simulink model "PlanarModelandControl"  (R2026a)
settings: Solver=FixedStepDiscrete  StopTime=StopTime  FixedStep=Ts  MaxStep=0  RelTol=1e-5  AbsTol=1e-3
PostLoadFcn:
  | Controles_Ativos = 1;
  | Vx_Ref = 0;
  | Vx_init = 0;
  | t_default = [0; 10000];
  | Brake_Cmd = [t_default, [0; 0]];
  | Accel_Cmd = [t_default, [0; 0]];
  | Steer_Cmd = [t_default, [0; 0]];
  | Flag_CruiseControl = 0;
  | Ts = 0.001;
  | load('TC_LUT.mat')
InitFcn:
  | % Carrega parâmetros gerais (Massa, Ganhos PID, Geometria)
  | run('Parameters.m');
  | 
  | % Carrega as Tabelas (Lookup Tables)
  | run('curva_steering.m');
  | run('curva_tq_motor.m');
  | load('TC_LUT.mat')
model workspace (MAT data in the model file):
  A = 1.22
  Ack = 1
  Cd = 1.1
  Cl = -2
  Cs = [19075.58 25992.8 31792.64 35898.02 39044.37]
  Fz0x = -811.9083
  Fz0y = -210.9467
  Fz_cs = [222.41 444.82 667.23 889.64 1112.05]
  IAY = 0
  IAx = 0
  IAy = 0
  Im = 0.00033
  It = 0.0094
  Iw = 0.1578
  Iw_ = 0.7
  Iz_car = 180
  K_50_understeer = 0.005184
  K_Stability = 1
  K_oversteer = -0.001
  Kappa_F = 0
  Kappa_R = 0
  Km_FM = 110000
  Km_Fm = 110000
  Km_RM = 120000
  Km_Rm = 120000
  KphiARB_F = 0
  KphiARB_R = 11000
  KphiF = 17054.5
  KphiR = 40034.8
  Kphim_F = 137500
  Kphim_R = 48600
  L = 1.53
  M = 300
  Mgt = 11.72
  P = 68.9476
  P0 = 55.4852
  R = 0.2032
  Rcurva = 20
  Re = 0.2
  SR = 3
  TV_On = 1
  Ts = 0.001
  W = 2940
  Wf = 308.034
  Wr = 339.426
  Ws = 2099.34
  YLL = 1.11022302463e-16
  cx1 = 1.3668
  cy1 = 1.839
  dpi = 0.242630467224
  dx1 = 2.1568
  dx2 = -0.065814
  dx3 = 0.0011254
  dy1 = 2.453
  dy2 = -0.11197
  dy3 = 10.2899
  ex1 = -0.19815
  ex2 = -0.62529
  ex3 = 1.3396
  ex4 = 0.0029464
  ey1 = 0.96085
  ey2 = -0.28662
  ey3 = 0.14389
  ey4 = 4.9274
  ey5 = -70.7165
  g = 9.81
  hcg = 0.252
  hcgs = 0.202
  hrc_f = 0.056
  hrc_r = 0.084
  hs_f = 0.222
  hs_r = 0.222
  hx1 = -0.003859
  hx2 = -0.000374
  hy1 = -0.000403
  hy2 = -0.000261
  kx1 = -36.9039
  kx2 = 22.3857
  kx3 = 0.61844
  ky1 = -83.2526
  ky2 = -5.565
  ky3 = 0.88491
  ky4 = 2.9994
  ky5 = -99.9393
  ky6 = -3.4705
  ky7 = -0.91452
  lf = 0.8415
  lr = 0.6885
  mass_FL = 67.5
  mass_FR = 67.5
  mass_RL = 82.5
  mass_RR = 82.5
  mi = 2
  ms = 240
  pty1 = 0.005744
  pty2 = 19.1334
  px1 = -0.039964
  px2 = -0.15015
  px3 = -0.32528
  px4 = -0.063273
  py1 = 0.38578
  py2 = 1.2048
  py3 = -0.14544
  py4 = 0.092886
  py5 = -0.82901
  r = 9
  rhoar = 1.22
  s_f = 1
  s_r = 0.9
  tf = 1.25
  tr = 1.25
  vx1 = -0.11922
  vx2 = 0.002205
  vy1 = 0.032907
  vy2 = 0.018068
  vy3 = -1.0429
  vy4 = 0.53712
  x_fl = 0.8415
  x_fr = 0.8415
  x_rl = 0.6885
  x_rr = 0.6885
  xs_f = 0.826
  xs_r = 0.704
  y_fl = 0.6
  y_fr = 0.6
  y_rl = 0.59
  y_rr = 0.59
values from neighbouring files (symbols this model uses):
  GG_mode = 0    [autocross.m]    (also in teste.mat)
  Gr = 11.72    [Parameters.m]    (also in teste.mat)
  K_variavel = [-0.001, 0, 0.0008, 0.0012, 0.0015]    [Parameters.m]    (also in teste.mat = [-0.001 0 0.0008 0.0012 0.0015])
  Ki_data = [283117.9493, 114831.615, 51596.0519, 30556.5344, 17525.221, 11773.8301, 10567.5001]*0.008    [Parameters.m]    (also in teste.mat = [2264.9435944 918.65292 412.7684152 244.4522752 140.201768 9)
  Kp_data = [2877.5702, 2303.9999, 1972.0835, 1556.8775, 1258.2451, 1022.7085, 982.7861, ]*5    [Parameters.m]    (also in teste.mat = [14387.851 11519.9995 9860.4175 7784.3875 6291.2255 5113.542)
  Mz_maxF = [0 66.3 132.6 198.9 265.2 330.23 396.52 462.83 529.112 595.42 661.73 728.03 794.33 860.62 926.92 993.23 1058.25 1124.55 1190.85 1257.15 1323.45 1389.75 1456.05 1522.35 1588.65 1654.95]    [Parameters.m]    (also in teste.mat = [0 66.3 132.6 198.9 265.2 330.23 396.52 462.83 529.112 595.4)
  Mz_minF = [0 -66.3 -132.6 -198.9 -265.2 -330.23 -396.52 -462.83 -529.112 -595.42 -661.73 -728.03 -794.33 -860.62 -926.92 -993.23 -1058.25 -1124.55 -1190.85 -1257.15 -1323.45 -1389.75 -1456.05 -1522.35 -1588.65 -1654.95]    [Parameters.m]    (also in teste.mat = [0 -66.3 -132.6 -198.9 -265.2 -330.23 -396.52 -462.83 -529.1)
  pedal = [0 4 8 12 16 20 24 28 32 36 40 44 48 52 56 60 64 68 72 76 80 84 88 92 96 100]    [Parameters.m]    (also in teste.mat = [0 4 8 12 16 20 24 28 32 36 40 44 ... (26 elements, 1x26)])
  rpm_355_novo = [0 10050 10100 10140 10170 10210 10250 10280 10320 10360         10390 10420 10460 10620 10770 10920 11050 11170 11280         11380 11470 11550 11625 11680 11730 11775 11790 11800]    [curva_tq_motor.m]    (also in teste.mat = [0 10050 10100 10140 10170 10210 10250 10280 10320 10360 103)
  Steering_inner = [-29.018 ... (first row only)    [curva_steering.m]    (also in teste.mat = [-29.018 -25.595 -22.357 -19.265 -16.292 -13.415 -10.619 -7.)
  Steering_outer = [-25.111 ... (first row only)    [curva_steering.m]    (also in teste.mat = [-25.111 -22.589 -20.082 -17.585 -15.094 -12.604 -10.11 -7.6)
  steering_vol = [-105 ... (first row only)    [curva_steering.m]    (also in teste.mat = [-105 -94.5 -84 -73.5 -63 -52.5 -42 -31.5 -21 -10.5 0 10.5 .)
  torque_355_novo = [16.2 16.2 16.0 15.8 15.6 15.4 15.2 15.0 14.8 14.6            14.4 14.2 14.0 13.0 12.0 11.0 10.0 9.0 8.0            7.0 6.0 5.0 4.0 3.0 2.0 1.0 0.5 0.0]    [curva_tq_motor.m]    (also in teste.mat = [16.2 16.2 16 15.8 15.6 15.4 15.2 15 14.8 14.6 14.4 14.2 ...)
  Vx = [0, 12.5, 23.6, 30, 35]    [Parameters.m]    (also in teste.mat = [0 12.5 23.6 30 35])
  Vx_axis = [5, 10, 15, 20, 25, 30, 35]    [Parameters.m]    (also in teste.mat = [5 10 15 20 25 30 35])
undefined symbols (not defined in the file or in its folder): DiscIntNeverNeededParam, magnitude
libraries: simulink x5, fuzblock x4, sl_discontinuities x3, simulink_extras x3, slpidlib x1
(part 2 of 4)

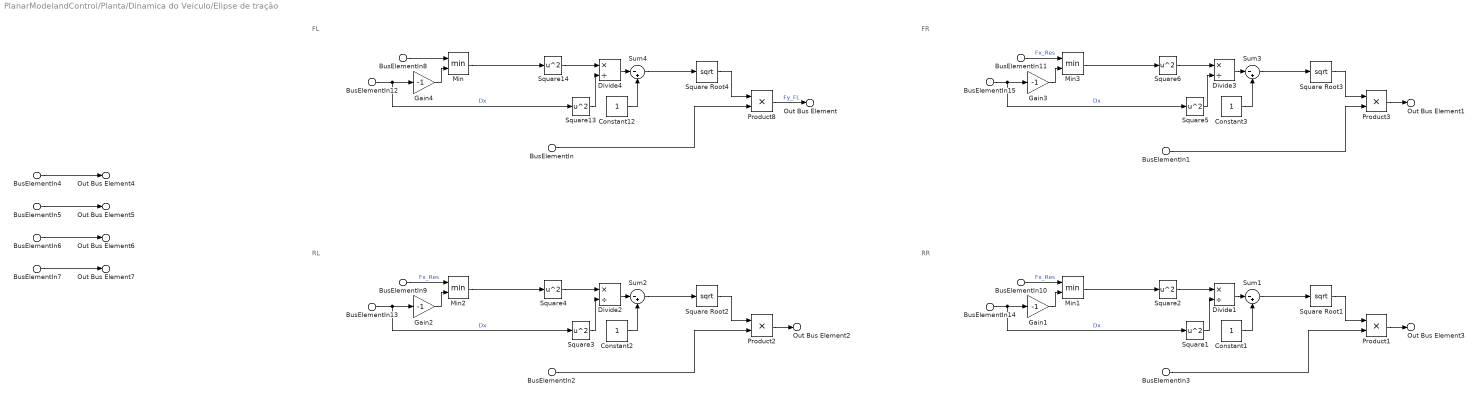
== system PlanarModelandControl/Planta/Dinamica do Veículo/Elipse de tração ==
  note: FL
  note: RR
  note: RL
  note: FR
  BusElementIn : Inport  PortName=Pacejka  Element=Fy0_FL
  BusElementIn1 : Inport  PortName=Pacejka  Element=Fy0_FR
  BusElementIn2 : Inport  PortName=Pacejka  Element=Fy0_RL
  BusElementIn3 : Inport  PortName=Pacejka  Element=Fy0_RR
  BusElementIn4 : Inport  PortName=Pacejka  Element=Fx0_FL
  BusElementIn8 : Inport  PortName=Pacejka  Element=Fx0_FL
  BusElementIn9 : Inport  PortName=Pacejka  Element=Fx0_RL
  BusElementIn6 : Inport  PortName=Pacejka  Element=Fx0_RL
  BusElementIn5 : Inport  PortName=Pacejka  Element=Fx0_FR
  BusElementIn11 : Inport  PortName=Pacejka  Element=Fx0_FR
  BusElementIn7 : Inport  PortName=Pacejka  Element=Fx0_RR
  BusElementIn10 : Inport  PortName=Pacejka  Element=Fx0_RR
  BusElementIn12 : Inport  PortName=Pacejka  Element=Dx_FL
  BusElementIn13 : Inport  PortName=Pacejka  Element=Dx_RL
  BusElementIn14 : Inport  PortName=Pacejka  Element=Dx_RR
  BusElementIn15 : Inport  PortName=Pacejka  Element=Dx_FR
  Constant{1..3,12} (4 alike) : Constant
  Divide{1..4} (4 alike) : Product  Inputs=*/
  Gain{1..4} (4 alike) : Gain  Gain=-1
  Min{,1..3} (4 alike) : MinMax  Inputs=2
  Product{1..3,8} (4 alike) : Product
  Square Root{1..4} (4 alike) : Sqrt
  Square{1..6,13..14} (8 alike) : Math  Operator=square
  Sum{1..4} (4 alike) : Sum  Inputs=|-+
  Out Bus Element : Outport  PortName=LatAndLongForces  Element=Fy_FL
  Out Bus Element3 : Outport  PortName=LatAndLongForces  Element=Fy_RR
  Out Bus Element2 : Outport  PortName=LatAndLongForces  Element=Fy_RL
  Out Bus Element1 : Outport  PortName=LatAndLongForces  Element=Fy_FR
  Out Bus Element4 : Outport  PortName=LatAndLongForces  Element=Fx_FL
  Out Bus Element5 : Outport  PortName=LatAndLongForces  Element=Fx_FR
  Out Bus Element6 : Outport  PortName=LatAndLongForces  Element=Fx_RL
  Out Bus Element7 : Outport  PortName=LatAndLongForces  Element=Fx_RR
  wires:
  Square13/1 -> Divide4/2
  Divide4/1 -> Sum4/1
  BusElementIn/1 -> Product8/2
  Square14/1 -> Divide4/1
  Constant12/1 -> Sum4/2
  BusElementIn8/1 -> Min/1
  Sum4/1 -> Square Root4/1
  BusElementIn12/1 -> Square13/1, Gain4/1  "Dx"
  Min/1 -> Square14/1
  Gain4/1 -> Min/2
  Product8/1 -> Out Bus Element/1  "Fy_FL"
  Square Root4/1 -> Product8/1
  BusElementIn10/1 -> Min1/1  "Fx_Res"
  Sum1/1 -> Square Root1/1
  Product1/1 -> Out Bus Element3/1
  Square2/1 -> Divide1/1
  Min1/1 -> Square2/1
  Constant1/1 -> Sum1/2
  Square1/1 -> Divide1/2
  Square Root1/1 -> Product1/1
  BusElementIn14/1 -> Square1/1, Gain1/1  "Dx"
  Gain1/1 -> Min1/2
  BusElementIn3/1 -> Product1/2
  Divide1/1 -> Sum1/1
  BusElementIn9/1 -> Min2/1  "Fx_Res"
  Sum2/1 -> Square Root2/1
  Product2/1 -> Out Bus Element2/1
  Square4/1 -> Divide2/1
  Min2/1 -> Square4/1
  Constant2/1 -> Sum2/2
  Square3/1 -> Divide2/2
  Square Root2/1 -> Product2/1
  BusElementIn13/1 -> Square3/1, Gain2/1  "Dx"
  Gain2/1 -> Min2/2
  BusElementIn2/1 -> Product2/2
  Divide2/1 -> Sum2/1
  BusElementIn11/1 -> Min3/1  "Fx_Res"
  Sum3/1 -> Square Root3/1
  Product3/1 -> Out Bus Element1/1
  Square6/1 -> Divide3/1
  Min3/1 -> Square6/1
  Constant3/1 -> Sum3/2
  Square5/1 -> Divide3/2
  Square Root3/1 -> Product3/1
  BusElementIn15/1 -> Square5/1, Gain3/1  "Dx"
  Gain3/1 -> Min3/2
  BusElementIn1/1 -> Product3/2
  Divide3/1 -> Sum3/1
  BusElementIn4/1 -> Out Bus Element4/1
  BusElementIn5/1 -> Out Bus Element5/1
  BusElementIn7/1 -> Out Bus Element7/1
  BusElementIn6/1 -> Out Bus Element6/1

== system PlanarModelandControl/Planta/Dinamica do Veículo/Frenagem ==
  Bus Element In6 : Inport  PortName=Driver  Element=BrakeCmd
  BusElementIn : Inport  Port=2  PortName=Movimento  Element=Vx_cg
  Constant : Constant  Value=0
  Gain : Gain  Gain=9508
  Switch : Switch  Criteria=u2 > Threshold  SaturateOnIntegerOverflow=off  InputSameDT=off
  Switch1 : Switch  Criteria=u2 > Threshold  SaturateOnIntegerOverflow=off  InputSameDT=off
  torque_máx_f : Gain  Gain=0.632
  torque_máx_r : Gain  Gain=1-0.632
  Bus Element Out2 : Outport  PortName=Brake  Element=Fbrake_FL
  Bus Element Out1 : Outport  PortName=Brake  Element=Fbrake_RL
  Bus Element Out3 : Outport  PortName=Brake  Element=Fbrake_FR
  Bus Element Out4 : Outport  PortName=Brake  Element=Fbrake_RR
  wires:
  torque_máx_r/1 -> Switch1/1
  Bus Element In6/1 -> Gain/1
  Constant/1 -> Switch1/3, Switch/3
  Switch1/1 -> Bus Element Out4/1, Bus Element Out1/1
  torque_máx_f/1 -> Switch/1
  Switch/1 -> Bus Element Out2/1, Bus Element Out3/1
  BusElementIn/1 -> Switch1/2, Switch/2
  Gain/1 -> torque_máx_r/1, torque_máx_f/1

== system PlanarModelandControl/Planta/Dinamica do Veículo/Load Transfer ==
  note: Lift
  In Bus Element : Inport  PortName=Movimento
  In Bus Element1 : Inport  PortName=Movimento
  Bus Element In5 : Inport  PortName=Movimento  Element=Vx_cg
  Gain10 : Gain  Gain=-1/2*rhoar*Cl*A
  Lateral Weight Transfer : Subsystem
  Longitudinal Weight Transfer : Subsystem
  Square : Math  Operator=square
  Total Normal Forces : Subsystem
  Out Bus Element : Outport  PortName=NormalForces
  wires:
  Square/1 -> Gain10/1
  Bus Element In5/1 -> Square/1
  In Bus Element/1 -> Longitudinal Weight Transfer/1(Movimento)
  In Bus Element1/1 -> Lateral Weight Transfer/1(Movimento)
  Longitudinal Weight Transfer/1(DeltaW_long) -> Total Normal Forces/2(DeltaW_long)
  Lateral Weight Transfer/1(DeltaW_lat) -> Total Normal Forces/3(DeltaW_lat)
  Gain10/1 -> Total Normal Forces/1(FLift)
  Total Normal Forces/1(NormalForces) -> Out Bus Element/1

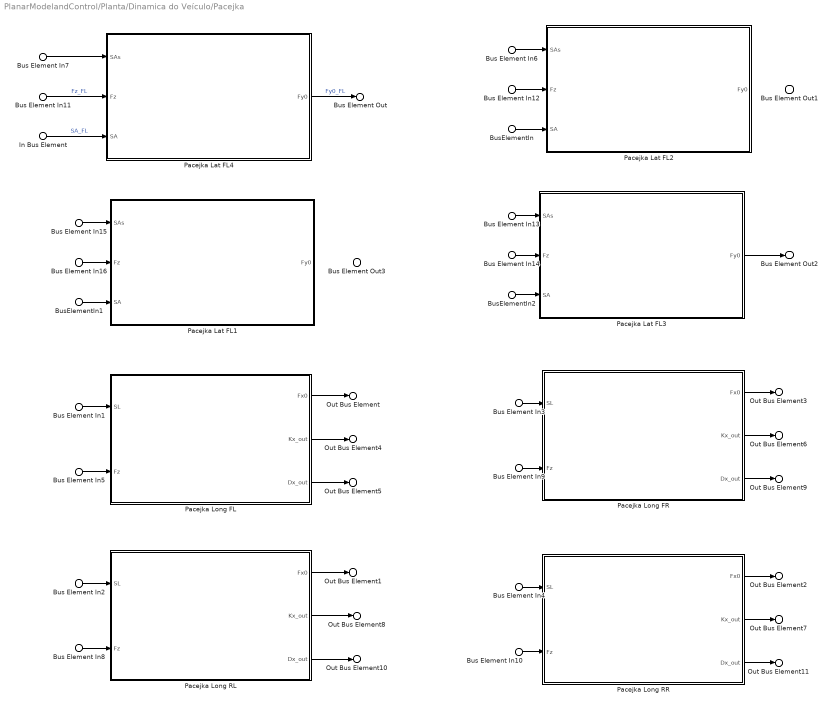
== system PlanarModelandControl/Planta/Dinamica do Veículo/Pacejka ==
  Bus Element In7 : Inport  PortName=RelaxionLength  Element=SAs_FL
  Bus Element In15 : Inport  PortName=RelaxionLength  Element=SAs_RL
  Bus Element In13 : Inport  PortName=RelaxionLength  Element=SAs_RR
  Bus Element In6 : Inport  PortName=RelaxionLength  Element=SAs_FR
  In Bus Element : Inport  Port=2  PortName=SlipAngles  Element=SA_FL
  BusElementIn : Inport  Port=2  PortName=SlipAngles  Element=SA_FR
  BusElementIn1 : Inport  Port=2  PortName=SlipAngles  Element=SA_RL
  BusElementIn2 : Inport  Port=2  PortName=SlipAngles  Element=SA_RR
  Bus Element In5 : Inport  Port=3  PortName=NormalForces  Element=Fz_FL
  Bus Element In11 : Inport  Port=3  PortName=NormalForces  Element=Fz_FL
  Bus Element In8 : Inport  Port=3  PortName=NormalForces  Element=Fz_RL
  Bus Element In16 : Inport  Port=3  PortName=NormalForces  Element=Fz_RL
  Bus Element In10 : Inport  Port=3  PortName=NormalForces  Element=Fz_RR
  Bus Element In14 : Inport  Port=3  PortName=NormalForces  Element=Fz_RR
  Bus Element In9 : Inport  Port=3  PortName=NormalForces  Element=Fz_FR
  Bus Element In12 : Inport  Port=3  PortName=NormalForces  Element=Fz_FR
  Bus Element In1 : Inport  Port=4  PortName=WheelSlips  Element=slip_long_FL
  Bus Element In3 : Inport  Port=4  PortName=WheelSlips  Element=slip_long_FR
  Bus Element In4 : Inport  Port=4  PortName=WheelSlips  Element=slip_long_RR
  Bus Element In2 : Inport  Port=4  PortName=WheelSlips  Element=slip_long_RL
  Pacejka Lat FL1 : Subsystem
  Pacejka Lat FL2 : Subsystem
  Pacejka Lat FL3 : Subsystem
  Pacejka Lat FL4 : Subsystem
  "Pacejka Long FL " : Subsystem
  Pacejka Long FR : Subsystem
  Pacejka Long RL : Subsystem
  Pacejka Long RR : Subsystem
  Bus Element Out : Outport  PortName=Pacejka  Element=Fy0_FL
  Out Bus Element : Outport  PortName=Pacejka  Element=Fx0_FL
  Out Bus Element1 : Outport  PortName=Pacejka  Element=Fx0_RL
  Out Bus Element2 : Outport  PortName=Pacejka  Element=Fx0_RR
  Out Bus Element3 : Outport  PortName=Pacejka  Element=Fx0_FR
  Out Bus Element4 : Outport  PortName=Pacejka  Element=Kx_FL
  Out Bus Element5 : Outport  PortName=Pacejka  Element=Dx_FL
  Out Bus Element8 : Outport  PortName=Pacejka  Element=Kx_RL
  Out Bus Element7 : Outport  PortName=Pacejka  Element=Kx_RR
  Out Bus Element6 : Outport  PortName=Pacejka  Element=Kx_FR
  Out Bus Element10 : Outport  PortName=Pacejka  Element=Dx_RL
  Out Bus Element11 : Outport  PortName=Pacejka  Element=Dx_RR
  Out Bus Element9 : Outport  PortName=Pacejka  Element=Dx_FR
  Bus Element Out2 : Outport  PortName=Pacejka  Element=Fy0_RR
  Bus Element Out1 : Outport  PortName=Pacejka  Element=Fy0_FR
  Bus Element Out3 : Outport  PortName=Pacejka  Element=Fy0_RL
  wires:
  Pacejka Lat FL4/1(Fy0) -> Bus Element Out/1  "Fy0_FL"
  "Pacejka Long FL "/1(Fx0) -> Out Bus Element/1
  Pacejka Long RL/1(Fx0) -> Out Bus Element1/1
  Pacejka Long RR/1(Fx0) -> Out Bus Element2/1
  Pacejka Long FR/1(Fx0) -> Out Bus Element3/1
  Bus Element In2/1 -> Pacejka Long RL/1(SL)
  Bus Element In3/1 -> Pacejka Long FR/1(SL)
  Bus Element In4/1 -> Pacejka Long RR/1(SL)
  Bus Element In1/1 -> "Pacejka Long FL "/1(SL)
  Bus Element In5/1 -> "Pacejka Long FL "/2(Fz)
  "Pacejka Long FL "/2(Kx_out) -> Out Bus Element4/1
  "Pacejka Long FL "/3(Dx_out) -> Out Bus Element5/1
  Bus Element In8/1 -> Pacejka Long RL/2(Fz)
  Bus Element In9/1 -> Pacejka Long FR/2(Fz)
  Bus Element In10/1 -> Pacejka Long RR/2(Fz)
  Pacejka Long FR/2(Kx_out) -> Out Bus Element6/1
  Pacejka Long RR/2(Kx_out) -> Out Bus Element7/1
  Pacejka Long RL/2(Kx_out) -> Out Bus Element8/1
  Pacejka Long FR/3(Dx_out) -> Out Bus Element9/1
  Pacejka Long RL/3(Dx_out) -> Out Bus Element10/1
  Pacejka Long RR/3(Dx_out) -> Out Bus Element11/1
  Bus Element In11/1 -> Pacejka Lat FL4/2(Fz)  "Fz_FL"
  Bus Element In7/1 -> Pacejka Lat FL4/1(SAs)
  Bus Element In12/1 -> Pacejka Lat FL2/2(Fz)
  ? -> Bus Element Out1/1
  Bus Element In6/1 -> Pacejka Lat FL2/1(SAs)
  Bus Element In14/1 -> Pacejka Lat FL3/2(Fz)
  Pacejka Lat FL3/1(Fy0) -> Bus Element Out2/1
  Bus Element In13/1 -> Pacejka Lat FL3/1(SAs)
  Bus Element In16/1 -> Pacejka Lat FL1/2(Fz)
  ? -> Bus Element Out3/1
  Bus Element In15/1 -> Pacejka Lat FL1/1(SAs)
  In Bus Element/1 -> Pacejka Lat FL4/3(SA)  "SA_FL"
  BusElementIn2/1 -> Pacejka Lat FL3/3(SA)
  BusElementIn/1 -> Pacejka Lat FL2/3(SA)
  BusElementIn1/1 -> Pacejka Lat FL1/3(SA)

== system PlanarModelandControl/Planta/Dinamica do Veículo/Relaxation Length ==
  note: Sigma - Relaxation Length
  Bus Element In1 : Inport  PortName=SlipAnges
  BusElementIn : Inport  Port=2  PortName=NormalForces  Element=Fz_FL
  BusElementIn1 : Inport  Port=2  PortName=NormalForces  Element=Fz_RL
  BusElementIn2 : Inport  Port=2  PortName=NormalForces  Element=Fz_RR
  BusElementIn3 : Inport  Port=2  PortName=NormalForces  Element=Fz_FR
  Bus Element In3 : Inport  Port=3  PortName=omega
  Atan{,1..3} (4 alike) : Trigonometry  Operator=atan
  Gain{,2,4,6} (4 alike) : Gain  Gain=1/(pty2*Fz0y)
  Gain{1,3,5,7} (4 alike) : Gain  Gain=2
  Gain{8..11} (4 alike) : Gain  Gain=pty1*Fz0y
  Sin{,1..3} (4 alike) : Trigonometry
  Subsystem : Subsystem
      settings: PortSchema="{\"entries\":[{\"content\":{\"sides\":[{\"content\":{\"connectorIds\":[\"In1\",\"In2\",\"In3\",\"In4\"]},\"type\":\"ConnectorPlacement.EquallySpacedRectSide\",\"uuid\":\"7f4a3b79-0cec-4aed-ad23-d33dc035a0be\"},{\"content\":{\"connectorIds\":[\"Out1\"],\"side\":\"RIGHT\"},\"type\":\"ConnectorPlacement.EquallySpacedRectSide\",\"uuid\":\"18b0376e-fcdd-4b64-8606-1eed368224ef\"},{\"content\":{\"connectorIds\":[\"In5\",\"In6\"],\"side\":\"TOP\"},\"type\":\"ConnectorPlacement.EquallySpacedRectSide\",\"uuid\":\"a3b43353-e9b4-4b33-921b-3423b398b207\"}]},\"type\":\"ConnectorPlacement.EquallySpacedConnector\",\"uuid\":\"fde8bdbb-5266-4a31-b332-536ff94eb04f\"}],\"packageUris\":[\"http://schema.mathworks.com/mf0/Connector/R2020b\"],\"version\":\"1.0\"}"
  Out Bus Element : Outport  PortName=RelaxionLength
  wires:
  Gain3/1 -> Sin1/1
  Gain4/1 -> Atan2/1
  Gain/1 -> Atan/1
  Gain5/1 -> Sin2/1
  Sin2/1 -> Gain10/1
  BusElementIn2/1 -> Gain6/1
  Gain2/1 -> Atan1/1
  Atan3/1 -> Gain7/1
  BusElementIn1/1 -> Gain4/1
  Gain6/1 -> Atan3/1
  Atan1/1 -> Gain3/1
  Gain1/1 -> Sin/1
  Gain7/1 -> Sin3/1
  Bus Element In3/1 -> Subsystem/6(omega)
  Gain11/1 -> Subsystem/4(RR)  "RR"
  Atan/1 -> Gain1/1
  Gain10/1 -> Subsystem/3(RL)  "RL"
  Gain8/1 -> Subsystem/1(FL)  "FL"
  Sin3/1 -> Gain11/1
  Gain9/1 -> Subsystem/2(FR)  "FR"
  Sin/1 -> Gain8/1
  BusElementIn/1 -> Gain/1
  Sin1/1 -> Gain9/1
  Atan2/1 -> Gain5/1
  BusElementIn3/1 -> Gain2/1
  Subsystem/1(RelaxionLength) -> Out Bus Element/1
  Bus Element In1/1 -> Subsystem/5(SlipAnges)

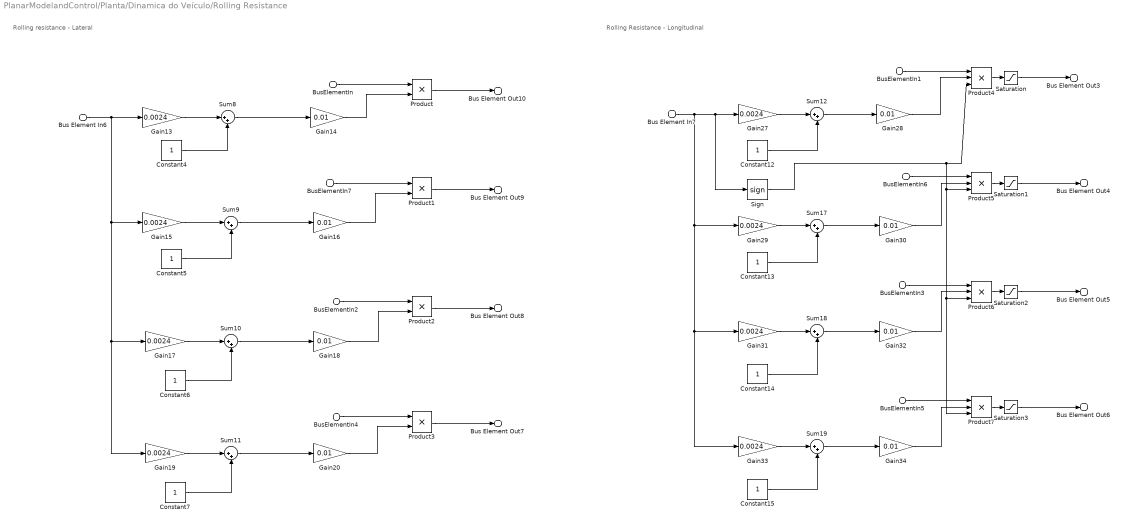
== system PlanarModelandControl/Planta/Dinamica do Veículo/Rolling Resistance ==
  note: Rolling Resistance - Longitudinal
  note: Rolling resistance - Lateral
  BusElementIn : Inport  PortName=NormalForces  Element=Fz_FL
  BusElementIn1 : Inport  PortName=NormalForces  Element=Fz_FL
  BusElementIn2 : Inport  PortName=NormalForces  Element=Fz_RL
  BusElementIn3 : Inport  PortName=NormalForces  Element=Fz_RL
  BusElementIn4 : Inport  PortName=NormalForces  Element=Fz_RR
  BusElementIn5 : Inport  PortName=NormalForces  Element=Fz_RR
  BusElementIn6 : Inport  PortName=NormalForces  Element=Fz_FR
  BusElementIn7 : Inport  PortName=NormalForces  Element=Fz_FR
  Bus Element In6 : Inport  Port=2  PortName=Movimento  Element=Vx_cg
  Bus Element In7 : Inport  Port=2  PortName=Movimento  Element=Vx_cg
  Constant{4..7,12..15} (8 alike) : Constant
  Gain{13,15,17,19,27,29,31,33} (8 alike) : Gain  Gain=0.0024
  Gain{14,16,18,20,28,30,32,34} (8 alike) : Gain  Gain=0.01
  Product{,1..3} (4 alike) : Product
  Product{4..7} (4 alike) : Product  Inputs=3
  Saturation{,1..3} (4 alike) : Saturate  UpperLimit=inf  LowerLimit=0
  Sign : Signum
  Sum{8..12,17..19} (8 alike) : Sum  Inputs=|++
  Bus Element Out3 : Outport  VectorParamsAs1DForOutWhenUnconnected=off  PortName=RollingResistances  Element=Frx_FL
  Bus Element Out4 : Outport  VectorParamsAs1DForOutWhenUnconnected=off  PortName=RollingResistances  Element=Frx_FR
  Bus Element Out5 : Outport  VectorParamsAs1DForOutWhenUnconnected=off  PortName=RollingResistances  Element=Frx_RL
  Bus Element Out6 : Outport  VectorParamsAs1DForOutWhenUnconnected=off  PortName=RollingResistances  Element=Frx_RR
  Bus Element Out7 : Outport  VectorParamsAs1DForOutWhenUnconnected=off  PortName=RollingResistances  Element=Fry_RR
  Bus Element Out8 : Outport  VectorParamsAs1DForOutWhenUnconnected=off  PortName=RollingResistances  Element=Fry_RL
  Bus Element Out9 : Outport  VectorParamsAs1DForOutWhenUnconnected=off  PortName=RollingResistances  Element=Fry_FR
  Bus Element Out10 : Outport  VectorParamsAs1DForOutWhenUnconnected=off  PortName=RollingResistances  Element=Fry_FL
  wires:
  Gain18/1 -> Product2/2
  Gain28/1 -> Product4/2
  BusElementIn5/1 -> Product7/1
  Product3/1 -> Bus Element Out7/1
  Gain34/1 -> Product7/2
  Constant4/1 -> Sum8/2
  Saturation/1 -> Bus Element Out3/1
  Gain14/1 -> Product/2
  Gain29/1 -> Sum17/1
  Sign/1 -> Product7/3, Product6/3, Product5/3, Product4/3
  Constant15/1 -> Sum19/2
  Product6/1 -> Saturation2/1
  Gain17/1 -> Sum10/1
  Saturation3/1 -> Bus Element Out6/1
  Sum8/1 -> Gain14/1
  Bus Element In7/1 -> Gain33/1, Gain31/1, Gain29/1, Sign/1, Gain27/1
  Sum11/1 -> Gain20/1
  Constant12/1 -> Sum12/2
  Saturation1/1 -> Bus Element Out4/1
  Saturation2/1 -> Bus Element Out5/1
  BusElementIn3/1 -> Product6/1
  Gain30/1 -> Product5/2
  Gain15/1 -> Sum9/1
  BusElementIn1/1 -> Product4/1
  Gain19/1 -> Sum11/1
  Sum12/1 -> Gain28/1
  Constant5/1 -> Sum9/2
  Sum9/1 -> Gain16/1
  Sum17/1 -> Gain30/1
  Product1/1 -> Bus Element Out9/1
  BusElementIn4/1 -> Product3/1
  Constant14/1 -> Sum18/2
  Sum10/1 -> Gain18/1
  Constant6/1 -> Sum10/2
  Constant7/1 -> Sum11/2
  Product2/1 -> Bus Element Out8/1
  Gain13/1 -> Sum8/1
  Bus Element In6/1 -> Gain19/1, Gain17/1, Gain15/1, Gain13/1
  Gain20/1 -> Product3/2
  Sum19/1 -> Gain34/1
  Product7/1 -> Saturation3/1
  Product/1 -> Bus Element Out10/1
  BusElementIn/1 -> Product/1
  Gain16/1 -> Product1/2
  Product5/1 -> Saturation1/1
  Gain31/1 -> Sum18/1
  Gain27/1 -> Sum12/1
  Sum18/1 -> Gain32/1
  Gain33/1 -> Sum19/1
  Constant13/1 -> Sum17/2
  Gain32/1 -> Product6/2
  BusElementIn2/1 -> Product2/1
  Product4/1 -> Saturation/1
  BusElementIn7/1 -> Product1/1
  BusElementIn6/1 -> Product5/1

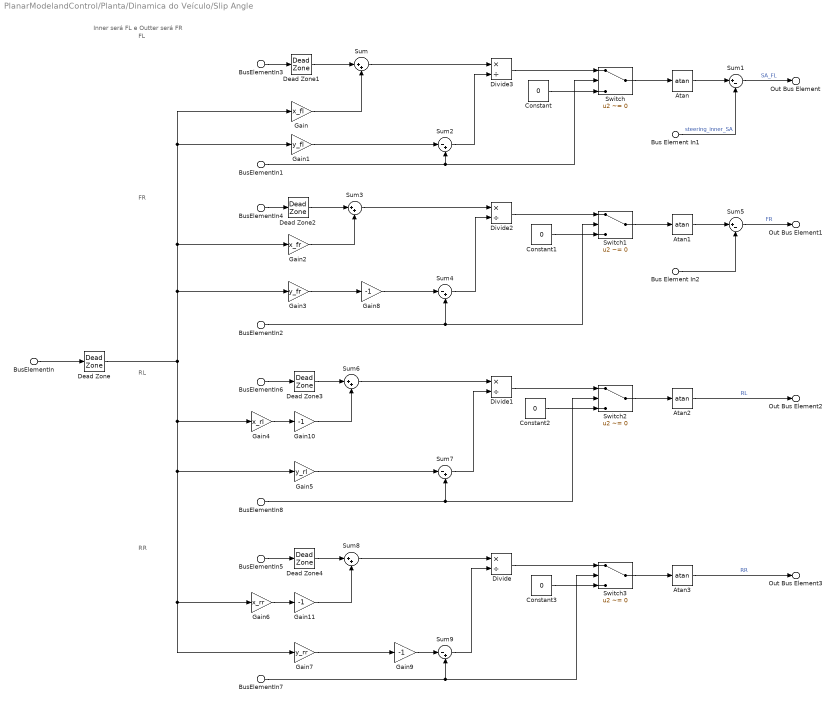
== system PlanarModelandControl/Planta/Dinamica do Veículo/Slip Angle ==
  note: FL
  note: FR
  note: Inner será FL e Outter será FR
  note: RL
  note: RR
  Bus Element In1 : Inport  PortName=Driver  Element=steering_inner
  Bus Element In2 : Inport  PortName=Driver  Element=steering_outer
  BusElementIn : Inport  Port=2  PortName=Movimento  Element=Yaw.v_yaw
  BusElementIn{1..2,7..8} (4 alike) : Inport  Port=2  PortName=Movimento  Element=Vx_cg
  BusElementIn{3..6} (4 alike) : Inport  Port=2  PortName=Movimento  Element=Vy_cg
  Atan{,1..3} (4 alike) : Trigonometry  Operator=atan
  Constant{,1..3} (4 alike) : Constant  Value=0
  Dead Zone{,1..4} (5 alike) : DeadZone  LowerValue=-0.01  UpperValue=0.01
  Divide{,1..3} (4 alike) : Product  Inputs=*/
  Gain : Gain  Gain=x_fl
  Gain1 : Gain  Gain=y_fl
  Gain{8..11} (4 alike) : Gain  Gain=-1
  Gain2 : Gain  Gain=x_fr
  Gain3 : Gain  Gain=y_fr
  Gain4 : Gain  Gain=x_rl
  Gain5 : Gain  Gain=y_rl
  Gain6 : Gain  Gain=x_rr
  Gain7 : Gain  Gain=y_rr
  Sum{,3,6,8} (4 alike) : Sum  Inputs=|++
  Sum1 : Sum  Inputs=|+-
  Sum{2,4,7,9} (4 alike) : Sum  Inputs=|-+
  Sum5 : Sum  Inputs=|+-
  Switch{,1..3} (4 alike) : Switch  Criteria=u2 ~= 0  SaturateOnIntegerOverflow=off  InputSameDT=off
  Out Bus Element : Outport  PortName=SlipAnges  Element=SA_FL
  Out Bus Element1 : Outport  PortName=SlipAnges  Element=SA_FR
  Out Bus Element2 : Outport  PortName=SlipAnges  Element=SA_RL
  Out Bus Element3 : Outport  PortName=SlipAnges  Element=SA_RR
  wires:
  Gain1/1 -> Sum2/1
  Sum3/1 -> Divide2/1
  Gain3/1 -> Gain8/1
  BusElementIn8/1 -> Sum7/2, Switch2/2
  Sum8/1 -> Divide/1
  BusElementIn7/1 -> Switch3/2, Sum9/2
  BusElementIn3/1 -> Dead Zone1/1
  BusElementIn4/1 -> Dead Zone2/1
  BusElementIn6/1 -> Dead Zone3/1
  BusElementIn5/1 -> Dead Zone4/1
  Gain4/1 -> Gain10/1
  Gain7/1 -> Gain9/1
  BusElementIn/1 -> Dead Zone/1
  Gain5/1 -> Sum7/1
  Gain9/1 -> Sum9/1
  Gain6/1 -> Gain11/1
  Gain/1 -> Sum/2
  Sum6/1 -> Divide1/1
  Gain8/1 -> Sum4/1
  Bus Element In1/1 -> Sum1/2  "steering_inner_SA"
  Bus Element In2/1 -> Sum5/2
  Sum/1 -> Divide3/1
  Atan/1 -> Sum1/1
  Atan1/1 -> Sum5/1
  Sum1/1 -> Out Bus Element/1  "SA_FL"
  Atan3/1 -> Out Bus Element3/1  "RR"
  Sum2/1 -> Divide3/2
  Divide3/1 -> Switch/1
  Constant/1 -> Switch/3
  Sum4/1 -> Divide2/2
  Divide2/1 -> Switch1/1
  Constant1/1 -> Switch1/3
  Switch1/1 -> Atan1/1
  Sum7/1 -> Divide1/2
  Divide1/1 -> Switch2/1
  Constant2/1 -> Switch2/3
  Switch2/1 -> Atan2/1
  Sum9/1 -> Divide/2
  Divide/1 -> Switch3/1
  Constant3/1 -> Switch3/3
  Switch3/1 -> Atan3/1
  Switch/1 -> Atan/1
  BusElementIn1/1 -> Sum2/2, Switch/2
  BusElementIn2/1 -> Sum4/2, Switch1/2
  Dead Zone/1 -> Gain4/1, Gain5/1, Gain6/1, Gain7/1, Gain3/1, Gain2/1, Gain1/1, Gain/1
  Dead Zone1/1 -> Sum/1
  Dead Zone2/1 -> Sum3/1
  Gain2/1 -> Sum3/2